simulink model "TosAeb"  (R2021a)
settings: Solver=FixedStepDiscrete  StartTime=0.0  StopTime=inf  FixedStep=0.01  RelTol=1e-3
undefined symbols (not defined in the file or in its folder): ACCModSts, AccSts1, AEB_ObjectMovingOrientation, AEB_ObjectMovingState, CN_BTCActive, CN_CurrentGearR, CN_CurrentGearR_DHT, CN_PTCActive, CN_VDCActive, CN_VSC_EspOff, CN_VSC_FCW_AEB_Error, CN_VSC_FCW_AEB_NoDispay, CN_VSC_FCW_AEB_PerfmDgdtn, CN_VSC_FCW_AEB_TempUnAvlbl, CN_VSC_VehStandstill, CNaccelAvoidMax, CNaccelAvoidMin, CNbrakePedalPress, CNcomfortStrLeftAccelMax, CNcomfortStrLeftAccelMin, CNcomfortStrRightAccelMax, CNcomfortStrRightAccelMin, CNDrvDoorOpen, CNDrvModeEco, CNDrvModeNorm, CNDrvModeOther, CNDrvModeSport, CNDrvSBRreminder, CNNull, CNobjInvalidID, CNPassDoorOpen, CNPassSBRreminder, CNPEBSAccelNegInf, CNPEBSAccelNull, CNPEBSTimeInf, CNPEBSTimeNull, CNSTOPMAX, CNSTOPNULL, CNsubjWidth, CNSwtOFF, CNSwtON, CNTTCMAX, CNTTCNULL, CNVehConfigAEBCycNone, CNVehConfigAEBNone, CNVehConfigAEBPedNone, CNVehConfigFCWNone, CNVehConfigJANone, CollisionSeverity, FALSE, FCWSnvty, JACycObjCalcInfoDefaultParams, JAPedObjCalcInfoDefaultParams, JAVehObjCalcInfoDefaultParams, latCycObjCalcInfoDefaultParams, latPedObjCalcInfoDefaultParams, longObjCalcInfoDefaultParams, ModSts, movingStatus, ObjectType ... (+1112)
libraries: simulink x3031, AEBSFunctionLib x50, Ta_Library x33, AEBSStateMachineLib x23, Mylibrary x12, simulink_extras x7, ObjSelect_lib x3, dspstat3 x2
(part 79 of 102)

== system TosAeb/FcwAebJa_Step_sys/ALG_AEBSystem/ALG_AEBController/AEB_LongTgtInfoCalc/LongObjCalcInfoCalc/AEBS_LongCollisionAssesApp/LongCollisionAvoidAssess/HighLvlBrkCalc/HighLvlBrkCalc4Brake/accelAvoidHighLvlBrkSituation3/If Action Subsystem1/DistReactionCalc ==
  subjLongAccel : Inport
  tReaction : Inport  Port=2
  subjVel : Inport  Port=3
  axAbsObj : Inport  Port=4
  vxAbsObj : Inport  Port=5
  dxFrontBumper : Inport  Port=6
  Abs : Abs
  Add : Sum
  Compare To Constant : simulink/Logic and Bit Operations/Compare To Constant  relop=<=  const=0.1  OutDataTypeStr=boolean
  Compare To Zero : simulink/Logic and Bit Operations/Compare To Zero  relop=>=  OutDataTypeStr=boolean
  Gain : Gain  Gain=0.5
  If : If  IfExpression=u1
  If Action Subsystem : Subsystem  TreatAsAtomicUnit=on
  If Action Subsystem1 : Subsystem  TreatAsAtomicUnit=on
  Math Function : Math  Operator=square  SignedPower=on  SaturateOnIntegerOverflow=on
  Merge : Merge
  Product : Product
  Product1 : Product
  Subtract : Sum  Inputs=+--
  Subtract1 : Sum  Inputs=-++
  collisionOcurredFlag : Outport
  dxRelTargetPredict : Outport  Port=2
  wires:
  If/1 -> If Action Subsystem/Action
  If Action Subsystem/1(dxObjReaction) -> Merge/1
  tReaction/1 -> Product/1, Math Function/1
  subjVel/1 -> Product/2
  subjLongAccel/1 -> Product1/1
  Compare To Constant/1 -> If/1
  Abs/1 -> Compare To Constant/1
  If/2 -> If Action Subsystem1/Action
  Product1/1 -> Gain/1
  Math Function/1 -> Product1/2
  Product/1 -> Add/2
  vxAbsObj/1 -> If Action Subsystem1/1(vxAbsObj)
  axAbsObj/1 -> Abs/1, If Action Subsystem1/2(axAbsObj)
  Gain/1 -> Add/1
  If Action Subsystem1/1(dxObjReaction) -> Merge/2
  Add/1 -> Subtract1/1, Subtract/1
  Merge/1 -> Subtract1/2, Subtract/2
  dxFrontBumper/1 -> Subtract1/3, Subtract/3
  Subtract/1 -> Compare To Zero/1
  Subtract1/1 -> dxRelTargetPredict/1
  Compare To Zero/1 -> collisionOcurredFlag/1

== system TosAeb/FcwAebJa_Step_sys/ALG_AEBSystem/ALG_AEBController/AEB_LongTgtInfoCalc/LongObjCalcInfoCalc/AEBS_LongCollisionAssesApp/LongCollisionAvoidAssess/HighLvlBrkCalc/HighLvlBrkCalc4Brake/accelAvoidHighLvlBrkSituation3/If Action Subsystem1/accelAvoidHighLvlBrkCalc ==
  subjLongAccel : Inport
  tReaction : Inport  Port=2
  subjVel : Inport  Port=3
  dxEgoBrake : Inport  Port=4
  Action Port : ActionPort  ActionPortLabel=else
  MovingSateCalcAfterReactionTime : Subsystem
  accelAvoidLowLvlBrkCalc : Subsystem  TreatAsAtomicUnit=on
  accelAvoidLowLvlBrkCalc1 : Subsystem  Commented=on  TreatAsAtomicUnit=on
  accelAvoidHighLvlBrk : Outport
  wires:
  tReaction/1 -> MovingSateCalcAfterReactionTime/2(tReaction)
  subjLongAccel/1 -> MovingSateCalcAfterReactionTime/1(subjLongAccel)
  subjVel/1 -> MovingSateCalcAfterReactionTime/3(subjVel)
  dxEgoBrake/1 -> accelAvoidLowLvlBrkCalc/6(dxEgoBrake)
  MovingSateCalcAfterReactionTime/4(subjVelPredict) -> accelAvoidLowLvlBrkCalc/4(subjVel)
  MovingSateCalcAfterReactionTime/2(axAbsTargetPredict) -> accelAvoidLowLvlBrkCalc/2(axAbsObj)
  MovingSateCalcAfterReactionTime/1(vxRelTargetPredict) -> accelAvoidLowLvlBrkCalc/1(vx)
  accelAvoidLowLvlBrkCalc/1(accelAvoidLowLvlBrk) -> accelAvoidHighLvlBrk/1
  MovingSateCalcAfterReactionTime/3(vxAbsTargetPredict) -> accelAvoidLowLvlBrkCalc/3(vxAbsObj)
  MovingSateCalcAfterReactionTime/5(tObjStopPredict) -> accelAvoidLowLvlBrkCalc/5(tObjStop)

== system TosAeb/FcwAebJa_Step_sys/ALG_AEBSystem/ALG_AEBController/AEB_LongTgtInfoCalc/LongObjCalcInfoCalc/AEBS_LongCollisionAssesApp/LongCollisionAvoidAssess/HighLvlBrkCalc/HighLvlBrkCalc4Brake/accelAvoidHighLvlBrkSituation5/If Action Subsystem1/CollisionJuge ==
  tReaction : Inport
  TTC : Inport  Port=2
  Relational Operator : RelationalOperator  Operator=>  OutDataTypeStr=boolean  RndMeth=Simplest
  collisonFlg : Outport
  wires:
  TTC/1 -> Relational Operator/2
  Relational Operator/1 -> collisonFlg/1
  tReaction/1 -> Relational Operator/1

== system TosAeb/FcwAebJa_Step_sys/ALG_AEBSystem/ALG_AEBController/AEB_LongTgtInfoCalc/LongObjCalcInfoCalc/AEBS_LongCollisionAssesApp/LongCollisionAvoidAssess/HighLvlBrkCalc/HighLvlBrkCalc4Brake/accelAvoidHighLvlBrkSituation5/If Action Subsystem1/If Action Subsystem ==
  Action Port : ActionPort  ActionPortLabel=if(u1)
  Constant : Constant  Value=CNPEBSAccelNegInf
  accelAvoidHighLvlBrk : Outport
  wires:
  Constant/1 -> accelAvoidHighLvlBrk/1

== system TosAeb/FcwAebJa_Step_sys/ALG_AEBSystem/ALG_AEBController/AEB_LongTgtInfoCalc/LongObjCalcInfoCalc/AEBS_LongCollisionAssesApp/LongCollisionAvoidAssess/HighLvlBrkCalc/HighLvlBrkCalc4Brake/accelAvoidHighLvlBrkSituation5/If Action Subsystem1/If Action Subsystem1 ==
  subjLongAccel : Inport
  tEgoStop : Inport  Port=2
  subjVel : Inport  Port=3
  axAbsObj : Inport  Port=4
  vxAbsObj : Inport  Port=5
  dxFrontBumper : Inport  Port=6
  Action Port : ActionPort  ActionPortLabel=else
  Abs : Abs
  Add1 : Sum
  Compare To Constant : simulink/Logic and Bit Operations/Compare To Constant  relop=<=  const=0.1  OutDataTypeStr=boolean
  Compare To Zero : simulink/Logic and Bit Operations/Compare To Zero  relop=<  OutDataTypeStr=boolean
  Constant : Constant  Value=CNaccelAvoidMin
  Constant1 : Constant  Value=CNaccelAvoidMax
  Gain1 : Gain  Gain=0.5
  If Action Subsystem2 : Subsystem  TreatAsAtomicUnit=on
  If Action Subsystem3 : Subsystem  TreatAsAtomicUnit=on
  If1 : If  IfExpression=u1
  Math Function1 : Math  Operator=square  SignedPower=on  SaturateOnIntegerOverflow=on
  Merge1 : Merge
  Product2 : Product
  Product3 : Product
  Subtract1 : Sum  Inputs=+--
  Switch : Switch  Criteria=u2 ~= 0
  accelAvoidHighLvlBrk : Outport
  wires:
  Switch/1 -> accelAvoidHighLvlBrk/1
  dxFrontBumper/1 -> Subtract1/3  "dRel"
  If1/1 -> If Action Subsystem2/Action
  subjVel/1 -> Product2/2
  If Action Subsystem3/1(dxObjReaction) -> Merge1/2
  Gain1/1 -> Add1/1
  Add1/1 -> Subtract1/1  "dEgo"
  If1/2 -> If Action Subsystem3/Action
  Abs/1 -> Compare To Constant/1
  Product2/1 -> Add1/2
  Compare To Constant/1 -> If1/1
  tEgoStop/1 -> Product2/1, Math Function1/1
  If Action Subsystem2/1(dxObjReaction) -> Merge1/1
  vxAbsObj/1 -> If Action Subsystem3/1(vxAbsObj)
  Product3/1 -> Gain1/1
  Math Function1/1 -> Product3/2
  axAbsObj/1 -> Abs/1, If Action Subsystem3/2(axAbsObj)
  subjLongAccel/1 -> Product3/1
  Merge1/1 -> Subtract1/2  "dObj"
  Subtract1/1 -> Compare To Zero/1
  Compare To Zero/1 -> Switch/2
  Constant1/1 -> Switch/1
  Constant/1 -> Switch/3

== system TosAeb/FcwAebJa_Step_sys/ALG_AEBSystem/ALG_AEBController/AEB_LongTgtInfoCalc/LongObjCalcInfoCalc/AEBS_LongCollisionAssesApp/LongCollisionAvoidAssess/HighLvlBrkCalc/HighLvlBrkCalc4Warning/accelAvoidHighLvlBrkSituation1/accelAvoidLowLvlBrkCalc/Situation1 ==
  dxEgoBrake : Inport
  vx : Inport  Port=2
  tObjStop : Inport  Port=3
  subjVel : Inport  Port=4
  vxAbsObj : Inport  Port=5
  axAbsObj : Inport  Port=6
  function : TriggerPort  TriggerType=function-call  VariantControl=Variant
  Bus Creator : BusCreator  Inputs=6
  Bus Selector : BusSelector  OutputSignals=dxEgoBrake,vx,tObjStop
  Bus Selector1 : BusSelector  OutputSignals=axAbsObj,vx,dxEgoBrake
  Bus Selector2 : BusSelector  OutputSignals=subjVel,vxAbsObj,axAbsObj,dxEgoBrake
  Case1 : Subsystem  TreatAsAtomicUnit=on
  Case2 : Subsystem  TreatAsAtomicUnit=on
  If : If  IfExpression=u1
  Merge : Merge
  collisionFirstFlgCalc : Subsystem
  accelAvoidLowLvlBrk : Outport
  wires:
  collisionFirstFlgCalc/1(collisionFirstFlg) -> If/1
  Bus Selector/3 -> collisionFirstFlgCalc/3(tObjStop)  "<tObjStop>"
  Bus Selector/2 -> collisionFirstFlgCalc/2(vx)  "<vx>"
  Bus Selector/1 -> collisionFirstFlgCalc/1(dxEgoBrake)  "<dxEgoBrake>"
  Bus Selector2/4 -> Case2/4(dxEgoBrake)  "<dxEgoBrake>"
  Bus Selector2/3 -> Case2/3(axAbsObj)  "<axAbsObj>"
  Bus Selector2/2 -> Case2/2(vxAbsObj)  "<vxAbsObj>"
  Bus Selector2/1 -> Case2/1(subjVel)  "<subjVel>"
  Bus Selector1/3 -> Case1/3(dxEgoBrake)  "<dxEgoBrake>"
  Bus Selector1/2 -> Case1/2(vx)  "<vx>"
  Bus Selector1/1 -> Case1/1(axAbsObj)  "<axAbsObj>"
  Bus Creator/1 -> Bus Selector/1, Bus Selector1/1, Bus Selector2/1
  vxAbsObj/1 -> Bus Creator/5  "vxAbsObj"
  axAbsObj/1 -> Bus Creator/6  "axAbsObj"
  dxEgoBrake/1 -> Bus Creator/1  "dxEgoBrake"
  subjVel/1 -> Bus Creator/4  "subjVel"
  vx/1 -> Bus Creator/2  "vx"
  tObjStop/1 -> Bus Creator/3  "tObjStop"
  [CASE2] -> Case2/Action
  If/2 -> [CASE2]
  Case2/1(accelAvoid) -> Merge/2
  Case1/1(accelAvoid) -> Merge/1
  Merge/1 -> accelAvoidLowLvlBrk/1
  If/1 -> Case1/Action

== system TosAeb/FcwAebJa_Step_sys/ALG_AEBSystem/ALG_AEBController/AEB_LongTgtInfoCalc/LongObjCalcInfoCalc/AEBS_LongCollisionAssesApp/LongCollisionAvoidAssess/HighLvlBrkCalc/HighLvlBrkCalc4Warning/accelAvoidHighLvlBrkSituation1/accelAvoidLowLvlBrkCalc/Situation2 ==
  axAbsObj : Inport
  vx : Inport  Port=2
  dxEgoBrake : Inport  Port=3
  function : TriggerPort  TriggerType=function-call  VariantControl=Variant
  Divide : Product  Inputs=*/
  Gain : Gain  Gain=2
  Math Function : Math  Operator=square  SignedPower=on  SaturateOnIntegerOverflow=on
  Subtract : Sum  Inputs=+-
  accelAvoidLowLvlBrk : Outport
  wires:
  Divide/1 -> Subtract/2
  Subtract/1 -> accelAvoidLowLvlBrk/1
  Gain/1 -> Divide/2
  dxEgoBrake/1 -> Gain/1
  Math Function/1 -> Divide/1
  vx/1 -> Math Function/1
  axAbsObj/1 -> Subtract/1

== system TosAeb/FcwAebJa_Step_sys/ALG_AEBSystem/ALG_AEBController/AEB_LongTgtInfoCalc/LongObjCalcInfoCalc/AEBS_LongCollisionAssesApp/LongCollisionAvoidAssess/HighLvlBrkCalc/HighLvlBrkCalc4Warning/accelAvoidHighLvlBrkSituation1/accelAvoidLowLvlBrkCalc/Situation3 ==
  note: aEgo^2
      | aAvoid = ----------------------------------
      | 		vObj^2
      | 		---------  - 2(dRel - dScure)
      | 		aObj
  subjVel : Inport
  vxAbsObj : Inport  Port=2
  axAbsObj : Inport  Port=3
  dxEgoBrake : Inport  Port=4
  function : TriggerPort  TriggerType=function-call  VariantControl=Variant
  Divide : Product  Inputs=*/
  Divide1 : Product  Inputs=*/
  Gain : Gain  Gain=2
  Math Function : Math  Operator=square  SignedPower=on  SaturateOnIntegerOverflow=on
  Math Function1 : Math  Operator=square  SignedPower=on  SaturateOnIntegerOverflow=on
  Subtract : Sum  Inputs=+-
  accelAvoidLowLvlBrk : Outport
  wires:
  Subtract/1 -> Divide1/2
  dxEgoBrake/1 -> Gain/1
  axAbsObj/1 -> Divide/2
  Divide/1 -> Subtract/1
  Math Function1/1 -> Divide1/1
  Divide1/1 -> accelAvoidLowLvlBrk/1
  Gain/1 -> Subtract/2
  Math Function/1 -> Divide/1
  subjVel/1 -> Math Function1/1
  vxAbsObj/1 -> Math Function/1

== system TosAeb/FcwAebJa_Step_sys/ALG_AEBSystem/ALG_AEBController/AEB_LongTgtInfoCalc/LongObjCalcInfoCalc/AEBS_LongCollisionAssesApp/LongCollisionAvoidAssess/HighLvlBrkCalc/HighLvlBrkCalc4Warning/accelAvoidHighLvlBrkSituation1/accelAvoidLowLvlBrkCalc/Situation4 ==
  function : TriggerPort  TriggerType=function-call  VariantControl=Variant
  Constant : Constant  Value=CNNull  VectorParams1D=off
  accelAvoidLowLvlBrk : Outport
  wires:
  Constant/1 -> accelAvoidLowLvlBrk/1

== system TosAeb/FcwAebJa_Step_sys/ALG_AEBSystem/ALG_AEBController/AEB_LongTgtInfoCalc/LongObjCalcInfoCalc/AEBS_LongCollisionAssesApp/LongCollisionAvoidAssess/HighLvlBrkCalc/HighLvlBrkCalc4Warning/accelAvoidHighLvlBrkSituation1/accelAvoidLowLvlBrkCalc/accelAvoidLowLvlBrkLimit ==
  accelAvoidLowLvlBrkNoLimit : Inport
  Saturation : Saturate  UpperLimit=CNNull  LowerLimit=CNaccelAvoidMin
      settings: AttributesFormatString=|
      | UpperLimit:%<UpperLimit>
      | LowerLimit:%<LowerLimit>
  accelAvoidLowLvlBrk : Outport
  wires:
  Saturation/1 -> accelAvoidLowLvlBrk/1
  accelAvoidLowLvlBrkNoLimit/1 -> Saturation/1

== system TosAeb/FcwAebJa_Step_sys/ALG_AEBSystem/ALG_AEBController/AEB_LongTgtInfoCalc/LongObjCalcInfoCalc/AEBS_LongCollisionAssesApp/LongCollisionAvoidAssess/HighLvlBrkCalc/HighLvlBrkCalc4Warning/accelAvoidHighLvlBrkSituation1/accelAvoidLowLvlBrkCalc2/Situation1 ==
  dxEgoBrake : Inport
  vx : Inport  Port=2
  tObjStop : Inport  Port=3
  subjVel : Inport  Port=4
  vxAbsObj : Inport  Port=5
  axAbsObj : Inport  Port=6
  function : TriggerPort  TriggerType=function-call  VariantControl=Variant
  Bus Creator : BusCreator  Inputs=6
  Bus Selector : BusSelector  OutputSignals=dxEgoBrake,vxRelTargetObjCalc,tObjStop
  Bus Selector1 : BusSelector  OutputSignals=axAbsTargetObjCalc,vxRelTargetObjCalc,dxEgoBrake
  Bus Selector2 : BusSelector  OutputSignals=subjVel,vxAbsTargetObjCalc,axAbsTargetObjCalc,dxEgoBrake
  Case1 : Subsystem  TreatAsAtomicUnit=on
  Case2 : Subsystem  TreatAsAtomicUnit=on
  If : If  IfExpression=u1
  Merge : Merge
  collisionFirstFlgCalc : Subsystem
  accelAvoidLowLvlBrk : Outport
  wires:
  If/1 -> Case1/Action
  Merge/1 -> accelAvoidLowLvlBrk/1
  Case1/1(accelAvoid) -> Merge/1
  Case2/1(accelAvoid) -> Merge/2
  If/2 -> [CASE2]
  [CASE2] -> Case2/Action
  tObjStop/1 -> Bus Creator/3  "tObjStop"
  vx/1 -> Bus Creator/2  "vxRelTargetObjCalc"
  subjVel/1 -> Bus Creator/4  "subjVel"
  dxEgoBrake/1 -> Bus Creator/1  "dxEgoBrake"
  axAbsObj/1 -> Bus Creator/6  "axAbsTargetObjCalc"
  vxAbsObj/1 -> Bus Creator/5  "vxAbsTargetObjCalc"
  Bus Creator/1 -> Bus Selector2/1, Bus Selector1/1, Bus Selector/1
  Bus Selector1/1 -> Case1/1(axAbsObj)  "<axAbsTargetObjCalc>"
  Bus Selector1/2 -> Case1/2(vx)  "<vxRelTargetObjCalc>"
  Bus Selector1/3 -> Case1/3(dxEgoBrake)  "<dxEgoBrake>"
  Bus Selector2/1 -> Case2/1(subjVel)  "<subjVel>"
  Bus Selector2/2 -> Case2/2(vxAbsObj)  "<vxAbsTargetObjCalc>"
  Bus Selector2/3 -> Case2/3(axAbsObj)  "<axAbsTargetObjCalc>"
  Bus Selector2/4 -> Case2/4(dxEgoBrake)  "<dxEgoBrake>"
  Bus Selector/1 -> collisionFirstFlgCalc/1(dxEgoBrake)  "<dxEgoBrake>"
  Bus Selector/2 -> collisionFirstFlgCalc/2(vx)  "<vxRelTargetObjCalc>"
  Bus Selector/3 -> collisionFirstFlgCalc/3(tObjStop)  "<tObjStop>"
  collisionFirstFlgCalc/1(collisionFirstFlg) -> If/1

== system TosAeb/FcwAebJa_Step_sys/ALG_AEBSystem/ALG_AEBController/AEB_LongTgtInfoCalc/LongObjCalcInfoCalc/AEBS_LongCollisionAssesApp/LongCollisionAvoidAssess/HighLvlBrkCalc/HighLvlBrkCalc4Warning/accelAvoidHighLvlBrkSituation1/accelAvoidLowLvlBrkCalc2/Situation2 ==
  axAbsObj : Inport
  vx : Inport  Port=2
  dxEgoBrake : Inport  Port=3
  function : TriggerPort  TriggerType=function-call  VariantControl=Variant
  Divide : Product  Inputs=*/
  Gain : Gain  Gain=2
  Math Function : Math  Operator=square  SignedPower=on  SaturateOnIntegerOverflow=on
  Subtract : Sum  Inputs=+-
  accelAvoidLowLvlBrk : Outport
  wires:
  axAbsObj/1 -> Subtract/1
  vx/1 -> Math Function/1
  Math Function/1 -> Divide/1
  dxEgoBrake/1 -> Gain/1
  Gain/1 -> Divide/2
  Subtract/1 -> accelAvoidLowLvlBrk/1
  Divide/1 -> Subtract/2

== system TosAeb/FcwAebJa_Step_sys/ALG_AEBSystem/ALG_AEBController/AEB_LongTgtInfoCalc/LongObjCalcInfoCalc/AEBS_LongCollisionAssesApp/LongCollisionAvoidAssess/HighLvlBrkCalc/HighLvlBrkCalc4Warning/accelAvoidHighLvlBrkSituation1/accelAvoidLowLvlBrkCalc2/Situation3 ==
  note: aEgo^2
      | aAvoid = ----------------------------------
      | 		vObj^2
      | 		---------  - 2(dRel - dScure)
      | 		aObj
  subjVel : Inport
  vxAbsObj : Inport  Port=2
  axAbsObj : Inport  Port=3
  dxEgoBrake : Inport  Port=4
  function : TriggerPort  TriggerType=function-call  VariantControl=Variant
  Divide : Product  Inputs=*/
  Divide1 : Product  Inputs=*/
  Gain : Gain  Gain=2
  Math Function : Math  Operator=square  SignedPower=on  SaturateOnIntegerOverflow=on
  Math Function1 : Math  Operator=square  SignedPower=on  SaturateOnIntegerOverflow=on
  Subtract : Sum  Inputs=+-
  accelAvoidLowLvlBrk : Outport
  wires:
  vxAbsObj/1 -> Math Function/1
  subjVel/1 -> Math Function1/1
  Math Function/1 -> Divide/1
  Gain/1 -> Subtract/2
  Divide1/1 -> accelAvoidLowLvlBrk/1
  Math Function1/1 -> Divide1/1
  Divide/1 -> Subtract/1
  axAbsObj/1 -> Divide/2
  dxEgoBrake/1 -> Gain/1
  Subtract/1 -> Divide1/2

== system TosAeb/FcwAebJa_Step_sys/ALG_AEBSystem/ALG_AEBController/AEB_LongTgtInfoCalc/LongObjCalcInfoCalc/AEBS_LongCollisionAssesApp/LongCollisionAvoidAssess/HighLvlBrkCalc/HighLvlBrkCalc4Warning/accelAvoidHighLvlBrkSituation1/accelAvoidLowLvlBrkCalc2/Situation4 ==
  function : TriggerPort  TriggerType=function-call  VariantControl=Variant
  Constant : Constant  Value=CNaccelAvoidMax  VectorParams1D=off
  accelAvoidLowLvlBrk : Outport
  wires:
  Constant/1 -> accelAvoidLowLvlBrk/1

== system TosAeb/FcwAebJa_Step_sys/ALG_AEBSystem/ALG_AEBController/AEB_LongTgtInfoCalc/LongObjCalcInfoCalc/AEBS_LongCollisionAssesApp/LongCollisionAvoidAssess/HighLvlBrkCalc/HighLvlBrkCalc4Warning/accelAvoidHighLvlBrkSituation1/accelAvoidLowLvlBrkCalc2/accelAvoidLowLvlBrkLimit ==
  accelAvoidLowLvlBrkNoLimit : Inport
  Saturation : Saturate  UpperLimit=CNaccelAvoidMax  LowerLimit=CNaccelAvoidMin
      settings: AttributesFormatString=|
      | UpperLimit:%<UpperLimit>
      | LowerLimit:%<LowerLimit>
  accelAvoidLowLvlBrk : Outport
  wires:
  accelAvoidLowLvlBrkNoLimit/1 -> Saturation/1
  Saturation/1 -> accelAvoidLowLvlBrk/1

== system TosAeb/FcwAebJa_Step_sys/ALG_AEBSystem/ALG_AEBController/AEB_LongTgtInfoCalc/LongObjCalcInfoCalc/AEBS_LongCollisionAssesApp/LongCollisionAvoidAssess/HighLvlBrkCalc/HighLvlBrkCalc4Warning/accelAvoidHighLvlBrkSituation2/accelAvoidHighLvlBrkCalc/If Action Subsystem ==
  Action Port : ActionPort  ActionPortLabel=if(u1 <= 0)
  Constant : Constant  Value=CNPEBSAccelNegInf
  accelAvoidHighLvlBrk : Outport
  wires:
  Constant/1 -> accelAvoidHighLvlBrk/1  "CNPEBSAccelNegInf"

== system TosAeb/FcwAebJa_Step_sys/ALG_AEBSystem/ALG_AEBController/AEB_LongTgtInfoCalc/LongObjCalcInfoCalc/AEBS_LongCollisionAssesApp/LongCollisionAvoidAssess/HighLvlBrkCalc/HighLvlBrkCalc4Warning/accelAvoidHighLvlBrkSituation2/accelAvoidHighLvlBrkCalc/If Action Subsystem1 ==
  vx : Inport
  axAbsObj : Inport  Port=2
  vxAbsObj : Inport  Port=3
  subjVel : Inport  Port=4
  tObjStop : Inport  Port=5
  dxEgoBrake : Inport  Port=6
  Action Port : ActionPort  ActionPortLabel=else
  accelAvoidLowLvlBrkCalc : Subsystem  TreatAsAtomicUnit=on
  accelAvoidLowLvlBrkCalc1 : Subsystem  Commented=on  TreatAsAtomicUnit=on
  accelAvoidHighLvlBrk : Outport
  wires:
  accelAvoidLowLvlBrkCalc/1(accelAvoidLowLvlBrk) -> accelAvoidHighLvlBrk/1
  dxEgoBrake/1 -> accelAvoidLowLvlBrkCalc/6(dxEgoBrake)
  tObjStop/1 -> accelAvoidLowLvlBrkCalc/5(tObjStop)
  subjVel/1 -> accelAvoidLowLvlBrkCalc/4(subjVel)
  vxAbsObj/1 -> accelAvoidLowLvlBrkCalc/3(vxAbsObj)
  axAbsObj/1 -> accelAvoidLowLvlBrkCalc/2(axAbsObj)
  vx/1 -> accelAvoidLowLvlBrkCalc/1(vx)

== system TosAeb/FcwAebJa_Step_sys/ALG_AEBSystem/ALG_AEBController/AEB_LongTgtInfoCalc/LongObjCalcInfoCalc/AEBS_LongCollisionAssesApp/LongCollisionAvoidAssess/HighLvlBrkCalc/HighLvlBrkCalc4Warning/accelAvoidHighLvlBrkSituation2/accelAvoidHighLvlBrkCalc/MovingSateCalcAfterReactionTime ==
  note: Both Ego and Obj keep moving after driver reaction time
  dxFrontBumper : Inport
  vx : Inport  Port=2
  tReaction : Inport  Port=3
  ax : Inport  Port=4
  subjLongAccel : Inport  Port=5
  subjVel : Inport  Port=6
  Add : Sum  Inputs=+++
  Add1 : Sum
  Add2 : Sum
  Add3 : Sum
  Constant : Constant  Value=CNNull
  Gain : Gain  Gain=0.5
  Math Function : Math  Operator=square  SignedPower=on  SaturateOnIntegerOverflow=on
  MinMax : MinMax  Function=max  Inputs=2
  Product{,1..3} (4 alike) : Product
  dxFrontBumperPredict : Outport
  vxPredict : Outport  Port=2
  vxAbsObjPredict : Outport  Port=3
  subjVelPredict : Outport  Port=4
  wires:
  dxFrontBumper/1 -> Add/1
  Add3/1 -> MinMax/1
  Math Function/1 -> Product/1
  subjLongAccel/1 -> Product3/2
  Add2/1 -> subjVelPredict/1, Add3/2  "subjVelPredict"
  Add/1 -> dxFrontBumperPredict/1  "dxFrontBumperPredict"
  Product3/1 -> Add2/1
  Product1/1 -> Add/2
  Gain/1 -> Add/3
  vx/1 -> Add1/2, Product1/1
  Product/1 -> Gain/1
  subjVel/1 -> Add2/2
  Add1/1 -> vxPredict/1, Add3/1  "vxPredict"
  ax/1 -> Product/2, Product2/2
  tReaction/1 -> Product1/2, Math Function/1, Product2/1, Product3/1
  Product2/1 -> Add1/1
  Constant/1 -> MinMax/2
  MinMax/1 -> vxAbsObjPredict/1

== system TosAeb/FcwAebJa_Step_sys/ALG_AEBSystem/ALG_AEBController/AEB_LongTgtInfoCalc/LongObjCalcInfoCalc/AEBS_LongCollisionAssesApp/LongCollisionAvoidAssess/HighLvlBrkCalc/HighLvlBrkCalc4Warning/accelAvoidHighLvlBrkSituation2/accelAvoidHighLvlBrkCalc/tObjStopCalc ==
  accelRel : Inport
  velRel : Inport  Port=2
  StopTimeCalc : Subsystem  TreatAsAtomicUnit=on
  StopTimeFlow : Stateflow chart  TreatAsAtomicUnit=on
  tStop : Outport
  wires:
  StopTimeFlow/2(tStopFuncCalc()) -> StopTimeCalc/Trigger
  StopTimeCalc/1(tStop) -> [tStopIn]  "tStopIn"
  [tStopIn] -> StopTimeFlow/3(tStopIn)
  StopTimeFlow/1(tStop) -> tStop/1  "tStop"
  accelRel/1 -> StopTimeCalc/2(accelRel), StopTimeFlow/1(accelRel)  "accelRel"
  velRel/1 -> StopTimeCalc/1(velRel), StopTimeFlow/2(velRel)  "velRel"

== system TosAeb/FcwAebJa_Step_sys/ALG_AEBSystem/ALG_AEBController/AEB_LongTgtInfoCalc/LongObjCalcInfoCalc/AEBS_LongCollisionAssesApp/LongCollisionAvoidAssess/HighLvlBrkCalc/HighLvlBrkCalc4Warning/accelAvoidHighLvlBrkSituation3/If Action Subsystem1/CollisionOcurredCase ==
  Action Port : ActionPort  ActionPortLabel=if(u1)
  Constant : Constant  Value=CNaccelAvoidMin
  accelAvoidHighLvlBrk : Outport
  wires:
  Constant/1 -> accelAvoidHighLvlBrk/1  "CNPEBSAccelNegInf"

== system TosAeb/FcwAebJa_Step_sys/ALG_AEBSystem/ALG_AEBController/AEB_LongTgtInfoCalc/LongObjCalcInfoCalc/AEBS_LongCollisionAssesApp/LongCollisionAvoidAssess/HighLvlBrkCalc/HighLvlBrkCalc4Warning/accelAvoidHighLvlBrkSituation3/If Action Subsystem1/DistReactionCalc ==
  subjLongAccel : Inport
  tReaction : Inport  Port=2
  subjVel : Inport  Port=3
  axAbsObj : Inport  Port=4
  vxAbsObj : Inport  Port=5
  dxFrontBumper : Inport  Port=6
  Abs : Abs
  Add : Sum
  Compare To Constant : simulink/Logic and Bit Operations/Compare To Constant  relop=<=  const=0.1  OutDataTypeStr=boolean
  Compare To Zero : simulink/Logic and Bit Operations/Compare To Zero  relop=>=  OutDataTypeStr=boolean
  Gain : Gain  Gain=0.5
  If : If  IfExpression=u1
  If Action Subsystem : Subsystem  TreatAsAtomicUnit=on
  If Action Subsystem1 : Subsystem  TreatAsAtomicUnit=on
  Math Function : Math  Operator=square  SignedPower=on  SaturateOnIntegerOverflow=on
  Merge : Merge
  Product : Product
  Product1 : Product
  Subtract : Sum  Inputs=+--
  Subtract1 : Sum  Inputs=-++
  collisionOcurredFlag : Outport
  dxRelTargetPredict : Outport  Port=2
  wires:
  Compare To Zero/1 -> collisionOcurredFlag/1
  Subtract1/1 -> dxRelTargetPredict/1
  Subtract/1 -> Compare To Zero/1
  dxFrontBumper/1 -> Subtract/3, Subtract1/3
  Merge/1 -> Subtract/2, Subtract1/2
  Add/1 -> Subtract/1, Subtract1/1
  If Action Subsystem1/1(dxObjReaction) -> Merge/2
  Gain/1 -> Add/1
  axAbsObj/1 -> If Action Subsystem1/2(axAbsObj), Abs/1
  vxAbsObj/1 -> If Action Subsystem1/1(vxAbsObj)
  Product/1 -> Add/2
  Math Function/1 -> Product1/2
  Product1/1 -> Gain/1
  If/2 -> If Action Subsystem1/Action
  Abs/1 -> Compare To Constant/1
  Compare To Constant/1 -> If/1
  subjLongAccel/1 -> Product1/1
  subjVel/1 -> Product/2
  tReaction/1 -> Math Function/1, Product/1
  If Action Subsystem/1(dxObjReaction) -> Merge/1
  If/1 -> If Action Subsystem/Action

== system TosAeb/FcwAebJa_Step_sys/ALG_AEBSystem/ALG_AEBController/AEB_LongTgtInfoCalc/LongObjCalcInfoCalc/AEBS_LongCollisionAssesApp/LongCollisionAvoidAssess/HighLvlBrkCalc/HighLvlBrkCalc4Warning/accelAvoidHighLvlBrkSituation3/If Action Subsystem1/accelAvoidHighLvlBrkCalc ==
  subjLongAccel : Inport
  tReaction : Inport  Port=2
  subjVel : Inport  Port=3
  dxEgoBrake : Inport  Port=4
  Action Port : ActionPort  ActionPortLabel=else
  MovingSateCalcAfterReactionTime : Subsystem
  accelAvoidLowLvlBrkCalc : Subsystem  TreatAsAtomicUnit=on
  accelAvoidLowLvlBrkCalc1 : Subsystem  Commented=on  TreatAsAtomicUnit=on
  accelAvoidHighLvlBrk : Outport
  wires:
  MovingSateCalcAfterReactionTime/5(tObjStopPredict) -> accelAvoidLowLvlBrkCalc/5(tObjStop)
  MovingSateCalcAfterReactionTime/3(vxAbsTargetPredict) -> accelAvoidLowLvlBrkCalc/3(vxAbsObj)
  accelAvoidLowLvlBrkCalc/1(accelAvoidLowLvlBrk) -> accelAvoidHighLvlBrk/1
  MovingSateCalcAfterReactionTime/1(vxRelTargetPredict) -> accelAvoidLowLvlBrkCalc/1(vx)
  MovingSateCalcAfterReactionTime/2(axAbsTargetPredict) -> accelAvoidLowLvlBrkCalc/2(axAbsObj)
  MovingSateCalcAfterReactionTime/4(subjVelPredict) -> accelAvoidLowLvlBrkCalc/4(subjVel)
  dxEgoBrake/1 -> accelAvoidLowLvlBrkCalc/6(dxEgoBrake)
  subjVel/1 -> MovingSateCalcAfterReactionTime/3(subjVel)
  subjLongAccel/1 -> MovingSateCalcAfterReactionTime/1(subjLongAccel)
  tReaction/1 -> MovingSateCalcAfterReactionTime/2(tReaction)

== system TosAeb/FcwAebJa_Step_sys/ALG_AEBSystem/ALG_AEBController/AEB_LongTgtInfoCalc/LongObjCalcInfoCalc/AEBS_LongCollisionAssesApp/LongCollisionAvoidAssess/HighLvlBrkCalc/HighLvlBrkCalc4Warning/accelAvoidHighLvlBrkSituation5/If Action Subsystem1/CollisionJuge ==
  tReaction : Inport
  TTC : Inport  Port=2
  Relational Operator : RelationalOperator  Operator=>  OutDataTypeStr=boolean  RndMeth=Simplest
  collisonFlg : Outport
  wires:
  tReaction/1 -> Relational Operator/1
  Relational Operator/1 -> collisonFlg/1
  TTC/1 -> Relational Operator/2

== system TosAeb/FcwAebJa_Step_sys/ALG_AEBSystem/ALG_AEBController/AEB_LongTgtInfoCalc/LongObjCalcInfoCalc/AEBS_LongCollisionAssesApp/LongCollisionAvoidAssess/HighLvlBrkCalc/HighLvlBrkCalc4Warning/accelAvoidHighLvlBrkSituation5/If Action Subsystem1/If Action Subsystem ==
  Action Port : ActionPort  ActionPortLabel=if(u1)
  Constant : Constant  Value=CNPEBSAccelNegInf
  accelAvoidHighLvlBrk : Outport
  wires:
  Constant/1 -> accelAvoidHighLvlBrk/1

== system TosAeb/FcwAebJa_Step_sys/ALG_AEBSystem/ALG_AEBController/AEB_LongTgtInfoCalc/LongObjCalcInfoCalc/AEBS_LongCollisionAssesApp/LongCollisionAvoidAssess/HighLvlBrkCalc/HighLvlBrkCalc4Warning/accelAvoidHighLvlBrkSituation5/If Action Subsystem1/If Action Subsystem1 ==
  subjLongAccel : Inport
  tEgoStop : Inport  Port=2
  subjVel : Inport  Port=3
  axAbsObj : Inport  Port=4
  vxAbsObj : Inport  Port=5
  dxFrontBumper : Inport  Port=6
  Action Port : ActionPort  ActionPortLabel=else
  Abs : Abs
  Add1 : Sum
  Compare To Constant : simulink/Logic and Bit Operations/Compare To Constant  relop=<=  const=0.1  OutDataTypeStr=boolean
  Compare To Zero : simulink/Logic and Bit Operations/Compare To Zero  relop=<  OutDataTypeStr=boolean
  Constant : Constant  Value=CNaccelAvoidMin
  Constant1 : Constant  Value=CNaccelAvoidMax
  Gain1 : Gain  Gain=0.5
  If Action Subsystem2 : Subsystem  TreatAsAtomicUnit=on
  If Action Subsystem3 : Subsystem  TreatAsAtomicUnit=on
  If1 : If  IfExpression=u1
  Math Function1 : Math  Operator=square  SignedPower=on  SaturateOnIntegerOverflow=on
  Merge1 : Merge
  Product2 : Product
  Product3 : Product
  Subtract1 : Sum  Inputs=+--
  Switch : Switch  Criteria=u2 ~= 0
  accelAvoidHighLvlBrk : Outport
  wires:
  Constant/1 -> Switch/3
  Constant1/1 -> Switch/1
  Compare To Zero/1 -> Switch/2
  Subtract1/1 -> Compare To Zero/1
  Merge1/1 -> Subtract1/2  "dObj"
  subjLongAccel/1 -> Product3/1
  axAbsObj/1 -> If Action Subsystem3/2(axAbsObj), Abs/1
  Math Function1/1 -> Product3/2
  Product3/1 -> Gain1/1
  vxAbsObj/1 -> If Action Subsystem3/1(vxAbsObj)
  If Action Subsystem2/1(dxObjReaction) -> Merge1/1
  tEgoStop/1 -> Math Function1/1, Product2/1
  Compare To Constant/1 -> If1/1
  Product2/1 -> Add1/2
  Abs/1 -> Compare To Constant/1
  If1/2 -> If Action Subsystem3/Action
  Add1/1 -> Subtract1/1  "dEgo"
  Gain1/1 -> Add1/1
  If Action Subsystem3/1(dxObjReaction) -> Merge1/2
  subjVel/1 -> Product2/2
  If1/1 -> If Action Subsystem2/Action
  dxFrontBumper/1 -> Subtract1/3  "dRel"
  Switch/1 -> accelAvoidHighLvlBrk/1

== system TosAeb/FcwAebJa_Step_sys/ALG_AEBSystem/ALG_AEBController/AEB_LongTgtInfoCalc/LongObjCalcInfoCalc/AEBS_LongCollisionAssesApp/TTCCalc/TTCCalc/Situation2/SloveTTCQuadraticEquation/AccelRelEqualsZero/Chart/TTCCalc ==
  distRel : Inport
  velRel : Inport  Port=2
  TTCCalc : TriggerPort  TriggerType=function-call  VariantControl=Variant
      settings: CopyFcn=Stateflow.SLINSF.SimulinkMan.removeBlockPreDeleteFcn  PreDeleteFcn=Stateflow.SLINSF.SimulinkMan.unsafePredeleteFcn
  Abs : Abs
  Abs1 : Abs
  Divide : Product  Inputs=*/
  tempTTC : Outport
  wires:
  Abs1/1 -> Divide/1
  Divide/1 -> tempTTC/1  "TTC"
  velRel/1 -> Abs/1  "velRel"
  distRel/1 -> Abs1/1  "distRel"
  Abs/1 -> Divide/2

== system TosAeb/FcwAebJa_Step_sys/ALG_AEBSystem/ALG_AEBController/AEB_LongTgtInfoCalc/LongObjCalcInfoCalc/AEBS_LongCollisionAssesApp/TTCCalc/TTCCalc/Situation2/SloveTTCQuadraticEquation/AccelRelUnequalZero/DeltaCalcu ==
  accelRel : Inport
  velRel : Inport  Port=2
  distRel : Inport  Port=3
  Gain : Gain  Gain=2
  Math Function : Math  Operator=square  SignedPower=on  SaturateOnIntegerOverflow=on
  Product : Product
  Subtract : Sum  Inputs=+-
  delta : Outport
  wires:
  distRel/1 -> Product/2  "distRel"
  Math Function/1 -> Subtract/1
  Subtract/1 -> delta/1  "delta"
  Gain/1 -> Subtract/2
  Product/1 -> Gain/1
  velRel/1 -> Math Function/1  "velRel"
  accelRel/1 -> Product/1  "accelRel"

== system TosAeb/FcwAebJa_Step_sys/ALG_AEBSystem/ALG_AEBController/AEB_LongTgtInfoCalc/LongObjCalcInfoCalc/AEBS_LongCollisionAssesApp/TTCCalc/TTCCalc/Situation2/SloveTTCQuadraticEquation/AccelRelUnequalZero/DeltaGreaterEqualZero ==
  delta : Inport
  velRel : Inport  Port=2
  accelRel : Inport  Port=3
  DeltaGreaterEqualZero : ActionPort  ActionPortLabel=if(u1 >= 0)
  Divide : Product  Inputs=*/
  Divide1 : Product  Inputs=*/
  Gain1 : Gain  Gain=-1
  RawTTCCalcu : Stateflow chart  TreatAsAtomicUnit=on
  Sqrt : Sqrt  SaturateOnIntegerOverflow=on
  Subtract1 : Sum  Inputs=+-
  Subtract2 : Sum
  TTC : Outport
  wires:
  Subtract1/1 -> Divide/1
  velRel/1 -> Gain1/1  "velRel"
  Subtract2/1 -> Divide1/1
  delta/1 -> Sqrt/1  "delta"
  Gain1/1 -> Subtract2/2, Subtract1/1
  accelRel/1 -> Divide/2, Divide1/2  "accelRel"
  Divide/1 -> RawTTCCalcu/1(TTC1)  "TTC1"
  Divide1/1 -> RawTTCCalcu/2(TTC2)  "TTC2"
  RawTTCCalcu/1(TTC) -> TTC/1  "TTC"
  Sqrt/1 -> Subtract2/1, Subtract1/2

== system TosAeb/FcwAebJa_Step_sys/ALG_AEBSystem/ALG_AEBController/AEB_LongTgtInfoCalc/LongObjCalcInfoCalc/AEBS_LongCollisionAssesApp/TTCCalc/TTCCalc/Situation2/SloveTTCQuadraticEquation/AccelRelUnequalZero/DeltaSmallerZero ==
  DeltaSmallerZero : ActionPort  ActionPortLabel=else
  Constant : Constant  Value=CNTTCMAX
  TTC : Outport
  wires:
  Constant/1 -> TTC/1  "TTC"

== system TosAeb/FcwAebJa_Step_sys/ALG_AEBSystem/ALG_AEBController/AEB_LongTgtInfoCalc/LongObjCalcInfoCalc/AEBS_LongCollisionAssesApp/TTCCalc/TTCCalc/Situation3/SloveTTCQuadraticEquation/AccelRelEqualsZero/Chart/TTCCalc ==
  distRel : Inport
  velRel : Inport  Port=2
  TTCCalc : TriggerPort  TriggerType=function-call  VariantControl=Variant
      settings: CopyFcn=Stateflow.SLINSF.SimulinkMan.removeBlockPreDeleteFcn  PreDeleteFcn=Stateflow.SLINSF.SimulinkMan.unsafePredeleteFcn
  Abs : Abs
  Abs1 : Abs
  Divide : Product  Inputs=*/
  tempTTC : Outport
  wires:
  Abs1/1 -> Divide/1
  Divide/1 -> tempTTC/1  "TTC"
  velRel/1 -> Abs/1  "velRel"
  distRel/1 -> Abs1/1  "distRel"
  Abs/1 -> Divide/2

== system TosAeb/FcwAebJa_Step_sys/ALG_AEBSystem/ALG_AEBController/AEB_LongTgtInfoCalc/LongObjCalcInfoCalc/AEBS_LongCollisionAssesApp/TTCCalc/TTCCalc/Situation3/SloveTTCQuadraticEquation/AccelRelUnequalZero/DeltaCalcu ==
  accelRel : Inport
  velRel : Inport  Port=2
  distRel : Inport  Port=3
  Gain : Gain  Gain=2
  Math Function : Math  Operator=square  SignedPower=on  SaturateOnIntegerOverflow=on
  Product : Product
  Subtract : Sum  Inputs=+-
  delta : Outport
  wires:
  distRel/1 -> Product/2  "distRel"
  Math Function/1 -> Subtract/1
  Subtract/1 -> delta/1  "delta"
  Gain/1 -> Subtract/2
  Product/1 -> Gain/1
  velRel/1 -> Math Function/1  "velRel"
  accelRel/1 -> Product/1  "accelRel"

== system TosAeb/FcwAebJa_Step_sys/ALG_AEBSystem/ALG_AEBController/AEB_LongTgtInfoCalc/LongObjCalcInfoCalc/AEBS_LongCollisionAssesApp/TTCCalc/TTCCalc/Situation3/SloveTTCQuadraticEquation/AccelRelUnequalZero/DeltaGreaterEqualZero ==
  delta : Inport
  velRel : Inport  Port=2
  accelRel : Inport  Port=3
  DeltaGreaterEqualZero : ActionPort  ActionPortLabel=if(u1 >= 0)
  Divide : Product  Inputs=*/
  Divide1 : Product  Inputs=*/
  Gain1 : Gain  Gain=-1
  RawTTCCalcu : Stateflow chart  TreatAsAtomicUnit=on
  Sqrt : Sqrt  SaturateOnIntegerOverflow=on
  Subtract1 : Sum  Inputs=+-
  Subtract2 : Sum
  TTC : Outport
  wires:
  Subtract1/1 -> Divide/1
  velRel/1 -> Gain1/1  "velRel"
  Subtract2/1 -> Divide1/1
  delta/1 -> Sqrt/1  "delta"
  Gain1/1 -> Subtract2/2, Subtract1/1
  accelRel/1 -> Divide/2, Divide1/2  "accelRel"
  Divide/1 -> RawTTCCalcu/1(TTC1)  "TTC1"
  Divide1/1 -> RawTTCCalcu/2(TTC2)  "TTC2"
  RawTTCCalcu/1(TTC) -> TTC/1  "TTC"
  Sqrt/1 -> Subtract2/1, Subtract1/2

== system TosAeb/FcwAebJa_Step_sys/ALG_AEBSystem/ALG_AEBController/AEB_LongTgtInfoCalc/LongObjCalcInfoCalc/AEBS_LongCollisionAssesApp/TTCCalc/TTCCalc/Situation3/SloveTTCQuadraticEquation/AccelRelUnequalZero/DeltaSmallerZero ==
  DeltaSmallerZero : ActionPort  ActionPortLabel=else
  Constant : Constant  Value=CNTTCMAX
  TTC : Outport
  wires:
  Constant/1 -> TTC/1  "TTC"

== system TosAeb/FcwAebJa_Step_sys/ALG_AEBSystem/ALG_AEBController/AEB_LongTgtInfoCalc/LongObjCalcInfoCalc/AEBS_LongCollisionAssesApp/collisionSeverityHighCalc/collisionSeverityHighCalc1/CollisionAssessHighCalcVersion3/CollisionAssessHighCalc1/CalcBrakePhase1/egoCollisionFlgCalc ==
  stEgo : Inport
  stObj : Inport  Port=2
  dx : Inport  Port=3
  Add : Sum
  Compare To Constant : simulink/Logic and Bit Operations/Compare To Constant  relop=<  const=0  OutDataTypeStr=boolean
  Subtract : Sum  Inputs=+-
  dxt : Outport
  egoCollisionFlg : Outport  Port=2
  wires:
  stObj/1 -> Add/1
  dx/1 -> Add/2
  Add/1 -> Subtract/1
  stEgo/1 -> Subtract/2
  Subtract/1 -> dxt/1, Compare To Constant/1
  Compare To Constant/1 -> egoCollisionFlg/1

== system TosAeb/FcwAebJa_Step_sys/ALG_AEBSystem/ALG_AEBController/AEB_LongTgtInfoCalc/LongObjCalcInfoCalc/AEBS_LongCollisionAssesApp/collisionSeverityHighCalc/collisionSeverityHighCalc1/CollisionAssessHighCalcVersion3/CollisionAssessHighCalc1/CalcBrakePhase1/restDeltaVelCalc ==
  velMaxReudce : Inport
  v0 : Inport  Port=2
  vt : Inport  Port=3
  Subtract : Sum  Inputs=+-
  Subtract1 : Sum  Inputs=+-
  restDeltaVel : Outport
  wires:
  v0/1 -> Subtract/1
  vt/1 -> Subtract/2
  Subtract/1 -> Subtract1/2
  velMaxReudce/1 -> Subtract1/1
  Subtract1/1 -> restDeltaVel/1

== system TosAeb/FcwAebJa_Step_sys/ALG_AEBSystem/ALG_AEBController/AEB_LongTgtInfoCalc/LongObjCalcInfoCalc/AEBS_LongCollisionAssesApp/collisionSeverityHighCalc/collisionSeverityHighCalc1/CollisionAssessHighCalcVersion3/CollisionAssessHighCalc1/CalcBrakePhase1/stEgoCalc ==
  tTime : Inport
  v0 : Inport  Port=2
  a0 : Inport  Port=3
  Add1 : Sum  Inputs=2
  Constant1 : Constant  Value=0.5
  Product1 : Product  Inputs=4
  Product4 : Product
  st : Outport
  wires:
  tTime/1 -> Product1/4, Product4/1, Product1/3
  Constant1/1 -> Product1/1
  a0/1 -> Product1/2
  v0/1 -> Product4/2
  Product1/1 -> Add1/1
  Product4/1 -> Add1/2
  Add1/1 -> st/1

== system TosAeb/FcwAebJa_Step_sys/ALG_AEBSystem/ALG_AEBController/AEB_LongTgtInfoCalc/LongObjCalcInfoCalc/AEBS_LongCollisionAssesApp/collisionSeverityHighCalc/collisionSeverityHighCalc1/CollisionAssessHighCalcVersion3/CollisionAssessHighCalc1/CalcBrakePhase1/stObjCalc ==
  tTime : Inport
  a0 : Inport  Port=2
  v0 : Inport  Port=3
  Add1 : Sum  Inputs=2
  Constant1 : Constant  Value=0.5
  Product1 : Product  Inputs=4
  Product4 : Product
  st : Outport
  wires:
  Add1/1 -> st/1
  Product4/1 -> Add1/2
  Product1/1 -> Add1/1
  v0/1 -> Product4/2
  a0/1 -> Product1/2
  Constant1/1 -> Product1/1
  tTime/1 -> Product4/1, Product1/4, Product1/3

== system TosAeb/FcwAebJa_Step_sys/ALG_AEBSystem/ALG_AEBController/AEB_LongTgtInfoCalc/LongObjCalcInfoCalc/AEBS_LongCollisionAssesApp/collisionSeverityHighCalc/collisionSeverityHighCalc1/CollisionAssessHighCalcVersion3/CollisionAssessHighCalc1/CalcBrakePhase1/tEgoStopCalc ==
  a0Ego : Inport
  v0Ego : Inport  Port=2
  tStopCalc : Subsystem  TreatAsAtomicUnit=on
  tEgoStop : Outport
  wires:
  v0Ego/1 -> tStopCalc/2(velAbs)
  a0Ego/1 -> tStopCalc/1(accelAbs)
  tStopCalc/1(tStop) -> tEgoStop/1

== system TosAeb/FcwAebJa_Step_sys/ALG_AEBSystem/ALG_AEBController/AEB_LongTgtInfoCalc/LongObjCalcInfoCalc/AEBS_LongCollisionAssesApp/collisionSeverityHighCalc/collisionSeverityHighCalc1/CollisionAssessHighCalcVersion3/CollisionAssessHighCalc1/CalcBrakePhase1/tObjRunCalc ==
  tEgoRun : Inport
  tObjStop : Inport  Port=2
  MinMax : MinMax  Inputs=2
  tObjRun : Outport
  wires:
  MinMax/1 -> tObjRun/1
  tEgoRun/1 -> MinMax/1
  tObjStop/1 -> MinMax/2

== system TosAeb/FcwAebJa_Step_sys/ALG_AEBSystem/ALG_AEBController/AEB_LongTgtInfoCalc/LongObjCalcInfoCalc/AEBS_LongCollisionAssesApp/collisionSeverityHighCalc/collisionSeverityHighCalc1/CollisionAssessHighCalcVersion3/CollisionAssessHighCalc1/CalcBrakePhase1/tObjStopCalc ==
  a0Obj : Inport
  v0Obj : Inport  Port=2
  tStopCalc : Subsystem  TreatAsAtomicUnit=on
  tObjStop : Outport
  wires:
  v0Obj/1 -> tStopCalc/2(velAbs)
  a0Obj/1 -> tStopCalc/1(accelAbs)
  tStopCalc/1(tStop) -> tObjStop/1

== system TosAeb/FcwAebJa_Step_sys/ALG_AEBSystem/ALG_AEBController/AEB_LongTgtInfoCalc/LongObjCalcInfoCalc/AEBS_LongCollisionAssesApp/collisionSeverityHighCalc/collisionSeverityHighCalc1/CollisionAssessHighCalcVersion3/CollisionAssessHighCalc1/CalcBrakePhase1/time4EgoPredic ==
  tEgoStop : Inport
  tEgovDelata : Inport  Port=2
  tBrakeLatency : Inport  Port=3
  MinMax : MinMax  Inputs=3
  tEgoRun : Outport
  wires:
  tBrakeLatency/1 -> MinMax/3
  tEgoStop/1 -> MinMax/1
  MinMax/1 -> tEgoRun/1
  tEgovDelata/1 -> MinMax/2

== system TosAeb/FcwAebJa_Step_sys/ALG_AEBSystem/ALG_AEBController/AEB_LongTgtInfoCalc/LongObjCalcInfoCalc/AEBS_LongCollisionAssesApp/collisionSeverityHighCalc/collisionSeverityHighCalc1/CollisionAssessHighCalcVersion3/CollisionAssessHighCalc1/CalcBrakePhase1/timeEgovDeltaCalc ==
  v0Ego : Inport
  velMaxReudce : Inport  Port=2
  a0Ego : Inport  Port=3
  timeEgovDelata : Subsystem  TreatAsAtomicUnit=on
  velMaxReudceFiltCalc : Subsystem
  tEgovDelata : Outport
  wires:
  timeEgovDelata/1(tStop) -> tEgovDelata/1
  a0Ego/1 -> timeEgovDelata/1(accelAbs)
  velMaxReudceFiltCalc/1(velMaxReudceFilt) -> timeEgovDelata/2(velAbs)
  velMaxReudce/1 -> velMaxReudceFiltCalc/2(velMaxReudce)
  v0Ego/1 -> velMaxReudceFiltCalc/1(v0Ego)

== system TosAeb/FcwAebJa_Step_sys/ALG_AEBSystem/ALG_AEBController/AEB_LongTgtInfoCalc/LongObjCalcInfoCalc/AEBS_LongCollisionAssesApp/collisionSeverityHighCalc/collisionSeverityHighCalc1/CollisionAssessHighCalcVersion3/CollisionAssessHighCalc1/CalcBrakePhase1/vtEgoCalc ==
  v0 : Inport
  a0 : Inport  Port=2
  tTime : Inport  Port=3
  Add1 : Sum
  Constant1 : Constant  Value=0
  MinMax : MinMax  Function=max  Inputs=2
  Product3 : Product
  vt : Outport
  wires:
  v0/1 -> Add1/2
  Product3/1 -> Add1/1
  a0/1 -> Product3/2
  tTime/1 -> Product3/1
  Add1/1 -> MinMax/1
  MinMax/1 -> vt/1
  Constant1/1 -> MinMax/2

== system TosAeb/FcwAebJa_Step_sys/ALG_AEBSystem/ALG_AEBController/AEB_LongTgtInfoCalc/LongObjCalcInfoCalc/AEBS_LongCollisionAssesApp/collisionSeverityHighCalc/collisionSeverityHighCalc1/CollisionAssessHighCalcVersion3/CollisionAssessHighCalc1/CalcBrakePhase1/vtObjCalc ==
  v0 : Inport
  a0 : Inport  Port=2
  tTime : Inport  Port=3
  Add1 : Sum
  Constant1 : Constant  Value=0
  MinMax : MinMax  Function=max  Inputs=2
  Product3 : Product
  vt : Outport
  wires:
  Constant1/1 -> MinMax/2
  MinMax/1 -> vt/1
  Add1/1 -> MinMax/1
  tTime/1 -> Product3/1
  a0/1 -> Product3/2
  Product3/1 -> Add1/1
  v0/1 -> Add1/2

== system TosAeb/FcwAebJa_Step_sys/ALG_AEBSystem/ALG_AEBController/AEB_LongTgtInfoCalc/LongObjCalcInfoCalc/AEBS_LongCollisionAssesApp/collisionSeverityHighCalc/collisionSeverityHighCalc1/CollisionAssessHighCalcVersion3/CollisionAssessHighCalc1/CalcBrakePhase2/BrkSysRuntimeFhase2 ==
  accelMaxDec : Inport
  a0Ego : Inport  Port=2
  accelGradient : Inport  Port=3
  If : If  IfExpression=u1
  If Action Subsystem : Subsystem  TreatAsAtomicUnit=on
  If Action Subsystem1 : Subsystem  TreatAsAtomicUnit=on
  Merge : Merge
  accelEgoLessAccelmaxReduce : Subsystem
  tTime : Outport
  wires:
  [InvalidCalc] -> If Action Subsystem1/Action
  If/2 -> [InvalidCalc]
  accelEgoLessAccelmaxReduce/1(Flg) -> If/1
  accelMaxDec/1 -> If Action Subsystem/2(accelMaxDec), accelEgoLessAccelmaxReduce/1(accelMaxDec)
  a0Ego/1 -> If Action Subsystem/1(a0Ego), accelEgoLessAccelmaxReduce/2(a0Ego)
  If/1 -> If Action Subsystem/Action
  accelGradient/1 -> If Action Subsystem/3(accelGradient)
  If Action Subsystem/1(tTime) -> Merge/1
  If Action Subsystem1/1(tTime) -> Merge/2
  Merge/1 -> tTime/1

== system TosAeb/FcwAebJa_Step_sys/ALG_AEBSystem/ALG_AEBController/AEB_LongTgtInfoCalc/LongObjCalcInfoCalc/AEBS_LongCollisionAssesApp/collisionSeverityHighCalc/collisionSeverityHighCalc1/CollisionAssessHighCalcVersion3/CollisionAssessHighCalc1/CalcBrakePhase2/Subsystem1 ==
  v0 : Inport
  a0 : Inport  Port=2
  accelGradient : Inport  Port=3
  tTime : Inport  Port=4
  Add : Sum
  Add1 : Sum
  Constant : Constant  Value=0.5
  Constant1 : Constant  Value=0
  MinMax : MinMax  Function=max  Inputs=2
  Product{,1..3} (4 alike) : Product
  vt : Outport
  wires:
  Constant1/1 -> MinMax/2
  MinMax/1 -> vt/1
  Add1/1 -> MinMax/1
  Constant/1 -> Product/1
  accelGradient/1 -> Product/2
  Product/1 -> Product1/1
  tTime/1 -> Product3/1, Product2/2, Product2/1
  Product2/1 -> Product1/2
  a0/1 -> Product3/2
  Product1/1 -> Add/1
  Product3/1 -> Add/2
  Add/1 -> Add1/1
  v0/1 -> Add1/2

== system TosAeb/FcwAebJa_Step_sys/ALG_AEBSystem/ALG_AEBController/AEB_LongTgtInfoCalc/LongObjCalcInfoCalc/AEBS_LongCollisionAssesApp/collisionSeverityHighCalc/collisionSeverityHighCalc1/CollisionAssessHighCalcVersion3/CollisionAssessHighCalc1/CalcBrakePhase2/egoCollisionFlgCalc ==
  stEgo : Inport
  stObj : Inport  Port=2
  dx : Inport  Port=3
  Add : Sum
  Compare To Constant : simulink/Logic and Bit Operations/Compare To Constant  relop=<  const=0  OutDataTypeStr=boolean
  Subtract : Sum  Inputs=+-
  dxt : Outport
  egoCollisionFlg : Outport  Port=2
  wires:
  stObj/1 -> Add/1
  dx/1 -> Add/2
  Add/1 -> Subtract/1
  stEgo/1 -> Subtract/2
  Subtract/1 -> dxt/1, Compare To Constant/1
  Compare To Constant/1 -> egoCollisionFlg/1

== system TosAeb/FcwAebJa_Step_sys/ALG_AEBSystem/ALG_AEBController/AEB_LongTgtInfoCalc/LongObjCalcInfoCalc/AEBS_LongCollisionAssesApp/collisionSeverityHighCalc/collisionSeverityHighCalc1/CollisionAssessHighCalcVersion3/CollisionAssessHighCalc1/CalcBrakePhase2/restDeltaVelCalc ==
  velMaxReudce : Inport
  v0Ego : Inport  Port=2
  vtEgo : Inport  Port=3
  Subtract : Sum  Inputs=+-
  Subtract1 : Sum  Inputs=+-
  restDeltaVelocity : Outport
  wires:
  Subtract1/1 -> restDeltaVelocity/1
  velMaxReudce/1 -> Subtract1/1
  Subtract/1 -> Subtract1/2
  vtEgo/1 -> Subtract/2
  v0Ego/1 -> Subtract/1

== system TosAeb/FcwAebJa_Step_sys/ALG_AEBSystem/ALG_AEBController/AEB_LongTgtInfoCalc/LongObjCalcInfoCalc/AEBS_LongCollisionAssesApp/collisionSeverityHighCalc/collisionSeverityHighCalc1/CollisionAssessHighCalcVersion3/CollisionAssessHighCalc1/CalcBrakePhase2/stEgoCalc ==
  tTime : Inport
  v0 : Inport  Port=2
  a0 : Inport  Port=3
  accelGradient : Inport  Port=4
  Add1 : Sum  Inputs=3
  Constant : Constant  Value=1/6
  Constant1 : Constant  Value=0.5
  Product : Product  Inputs=5
  Product1 : Product  Inputs=4
  Product4 : Product
  st : Outport
  wires:
  Add1/1 -> st/1
  Product4/1 -> Add1/3
  Product1/1 -> Add1/2
  Product/1 -> Add1/1
  v0/1 -> Product4/2
  a0/1 -> Product1/3
  Constant1/1 -> Product1/4
  Constant/1 -> Product/1
  accelGradient/1 -> Product/2
  tTime/1 -> Product/3, Product/5, Product1/2, Product4/1, Product1/1, Product/4

== system TosAeb/FcwAebJa_Step_sys/ALG_AEBSystem/ALG_AEBController/AEB_LongTgtInfoCalc/LongObjCalcInfoCalc/AEBS_LongCollisionAssesApp/collisionSeverityHighCalc/collisionSeverityHighCalc1/CollisionAssessHighCalcVersion3/CollisionAssessHighCalc1/CalcBrakePhase2/stObjCalc ==
  tTime : Inport
  a0 : Inport  Port=2
  v0 : Inport  Port=3
  Add1 : Sum  Inputs=2
  Constant1 : Constant  Value=0.5
  Product1 : Product  Inputs=4
  Product4 : Product
  st : Outport
  wires:
  Add1/1 -> st/1
  Product4/1 -> Add1/2
  Product1/1 -> Add1/1
  v0/1 -> Product4/2
  a0/1 -> Product1/2
  Constant1/1 -> Product1/1
  tTime/1 -> Product4/1, Product1/4, Product1/3

== system TosAeb/FcwAebJa_Step_sys/ALG_AEBSystem/ALG_AEBController/AEB_LongTgtInfoCalc/LongObjCalcInfoCalc/AEBS_LongCollisionAssesApp/collisionSeverityHighCalc/collisionSeverityHighCalc1/CollisionAssessHighCalcVersion3/CollisionAssessHighCalc1/CalcBrakePhase2/tEgoStopCalc ==
  a0Ego : Inport
  v0Ego : Inport  Port=2
  tStopCalc : Subsystem  TreatAsAtomicUnit=on
  tEgoStop : Outport
  wires:
  v0Ego/1 -> tStopCalc/2(velAbs)
  a0Ego/1 -> tStopCalc/1(accelAbs)
  tStopCalc/1(tStop) -> tEgoStop/1

== system TosAeb/FcwAebJa_Step_sys/ALG_AEBSystem/ALG_AEBController/AEB_LongTgtInfoCalc/LongObjCalcInfoCalc/AEBS_LongCollisionAssesApp/collisionSeverityHighCalc/collisionSeverityHighCalc1/CollisionAssessHighCalcVersion3/CollisionAssessHighCalc1/CalcBrakePhase2/tObjRunCalc ==
  tEgoRun : Inport
  tObjStop : Inport  Port=2
  MinMax : MinMax  Inputs=2
  tObjRun : Outport
  wires:
  MinMax/1 -> tObjRun/1
  tEgoRun/1 -> MinMax/1
  tObjStop/1 -> MinMax/2

== system TosAeb/FcwAebJa_Step_sys/ALG_AEBSystem/ALG_AEBController/AEB_LongTgtInfoCalc/LongObjCalcInfoCalc/AEBS_LongCollisionAssesApp/collisionSeverityHighCalc/collisionSeverityHighCalc1/CollisionAssessHighCalcVersion3/CollisionAssessHighCalc1/CalcBrakePhase2/tObjStopCalc ==
  a0Obj : Inport
  v0Obj : Inport  Port=2
  tStopCalc : Subsystem  TreatAsAtomicUnit=on
  tObjStop : Outport
  wires:
  v0Obj/1 -> tStopCalc/2(velAbs)
  a0Obj/1 -> tStopCalc/1(accelAbs)
  tStopCalc/1(tStop) -> tObjStop/1

== system TosAeb/FcwAebJa_Step_sys/ALG_AEBSystem/ALG_AEBController/AEB_LongTgtInfoCalc/LongObjCalcInfoCalc/AEBS_LongCollisionAssesApp/collisionSeverityHighCalc/collisionSeverityHighCalc1/CollisionAssessHighCalcVersion3/CollisionAssessHighCalc1/CalcBrakePhase2/time4EgoPredic ==
  tEgoStop : Inport
  tEgovDelata : Inport  Port=2
  tTime : Inport  Port=3
  MinMax : MinMax  Inputs=3
  tEgoRun : Outport
  wires:
  tTime/1 -> MinMax/3
  tEgoStop/1 -> MinMax/1
  MinMax/1 -> tEgoRun/1
  tEgovDelata/1 -> MinMax/2

== system TosAeb/FcwAebJa_Step_sys/ALG_AEBSystem/ALG_AEBController/AEB_LongTgtInfoCalc/LongObjCalcInfoCalc/AEBS_LongCollisionAssesApp/collisionSeverityHighCalc/collisionSeverityHighCalc1/CollisionAssessHighCalcVersion3/CollisionAssessHighCalc1/CalcBrakePhase2/timeEgovDeltaCalc ==
  v0Ego : Inport
  velMaxReudce : Inport  Port=2
  a0Ego : Inport  Port=3
  timeEgovDelata : Subsystem  TreatAsAtomicUnit=on
  velMaxReudceFiltCalc : Subsystem
  tEgovDelata : Outport
  wires:
  timeEgovDelata/1(tStop) -> tEgovDelata/1
  a0Ego/1 -> timeEgovDelata/1(accelAbs)
  velMaxReudceFiltCalc/1(velMaxReudceFilt) -> timeEgovDelata/2(velAbs)
  velMaxReudce/1 -> velMaxReudceFiltCalc/2(velMaxReudce)
  v0Ego/1 -> velMaxReudceFiltCalc/1(v0Ego)

== system TosAeb/FcwAebJa_Step_sys/ALG_AEBSystem/ALG_AEBController/AEB_LongTgtInfoCalc/LongObjCalcInfoCalc/AEBS_LongCollisionAssesApp/collisionSeverityHighCalc/collisionSeverityHighCalc1/CollisionAssessHighCalcVersion3/CollisionAssessHighCalc1/CalcBrakePhase2/vtObjCalc ==
  v0 : Inport
  a0 : Inport  Port=2
  tTime : Inport  Port=3
  Add1 : Sum
  Constant1 : Constant  Value=0
  MinMax : MinMax  Function=max  Inputs=2
  Product3 : Product
  vt : Outport
  wires:
  Constant1/1 -> MinMax/2
  MinMax/1 -> vt/1
  Add1/1 -> MinMax/1
  tTime/1 -> Product3/1
  a0/1 -> Product3/2
  Product3/1 -> Add1/1
  v0/1 -> Add1/2
